simulink model "MX_Gateway"  (Simulink 9.0) (continued)
  CD_EOT_DEBOUNCE_LOW = Bobcat.Parameter; CreateAliasCD( CD_EOT_DEBOUNCE_LOW                                  ,uint16(2)                  ,'ConstantDataPointer' ,'CONSTANT_DATA' ,'EOT_DEBOUNCE_LOW')    [cdata.m]    (also in hc12_cdata.m; m4_cdata.m)
  CD_EOT_DEBOUNCE_NORMAL = Bobcat.Parameter; CreateAliasCD( CD_EOT_DEBOUNCE_NORMAL                               ,uint16(2)                  ,'ConstantDataPointer' ,'CONSTANT_DATA' ,'EOT_DEBOUNCE_NORMAL')    [cdata.m]    (also in hc12_cdata.m; m4_cdata.m)
  CD_EOT_EXTREMELY_LOW = Bobcat.Parameter; CreateAliasCD( CD_EOT_EXTREMELY_LOW                                 ,uint16(81)                 ,'ConstantDataPointer' ,'CONSTANT_DATA' ,'EOT_EXTREMELY_LOW')    [cdata.m]    (also in hc12_cdata.m; m4_cdata.m)
  CD_EOT_FUNCTION = Bobcat.Parameter; CreateAliasCD( CD_EOT_FUNCTION                                      ,uint8(255)                 ,'ConstantDataPointer' ,'CONSTANT_DATA' ,'EOT_FUNCTION')    [cdata.m]    (also in hc12_cdata.m; m4_cdata.m)
  CD_EOT_LOW = Bobcat.Parameter; CreateAliasCD( CD_EOT_LOW                                           ,uint16(90)                 ,'ConstantDataPointer' ,'CONSTANT_DATA' ,'EOT_LOW')    [cdata.m]    (also in hc12_cdata.m; m4_cdata.m)
  CD_EOT_TEMPERATURE_HYSTERESIS = Bobcat.Parameter; CreateAliasCD( CD_EOT_TEMPERATURE_HYSTERESIS                        ,uint16(2)                  ,'ConstantDataPointer' ,'CONSTANT_DATA' ,'EOT_TEMPERATURE_HYSTERESIS')    [cdata.m]    (also in hc12_cdata.m; m4_cdata.m)
  CD_FAST_TRAVEL_FUNCTION = Bobcat.Parameter; CreateAliasCD( CD_FAST_TRAVEL_FUNCTION                              ,uint8(255)                 ,'ConstantDataPointer' ,'CONSTANT_DATA' ,'FAST_TRAVEL_FUNCTION')    [cdata.m]    (also in hc12_cdata.m; m4_cdata.m)
  CD_FAST_TRAVEL_THROTTLE_DELAY = Bobcat.Parameter; CreateAliasCD( CD_FAST_TRAVEL_THROTTLE_DELAY                        ,uint16(10)                 ,'ConstantDataPointer' ,'CONSTANT_DATA' ,'FAST_TRAVEL_THROTTLE_DELAY')    [cdata.m]    (also in hc12_cdata.m; m4_cdata.m)
  CD_FAST_TRAVEL_THROTTLE_LIMIT = Bobcat.Parameter; CreateAliasCD( CD_FAST_TRAVEL_THROTTLE_LIMIT                        ,uint16(1000)               ,'ConstantDataPointer' ,'CONSTANT_DATA' ,'FAST_TRAVEL_THROTTLE_LIMIT')    [cdata.m]    (also in hc12_cdata.m; m4_cdata.m)
  CD_FLYWHEEL_TEETH = Bobcat.Parameter; CreateAliasCD( CD_FLYWHEEL_TEETH                                    ,uint8(83)                  ,'ConstantDataPointer' ,'CONSTANT_DATA' ,'FLYWHEEL_TEETH')    [cdata.m]    (also in hc12_cdata.m; m4_cdata.m)
  CD_FUEL_LEVEL_FILTER_FAST_VALUE = Bobcat.Parameter; CreateAliasCD( CD_FUEL_LEVEL_FILTER_FAST_VALUE                      ,uint8(5)                   ,'ConstantDataPointer' ,'CONSTANT_DATA' ,'FUEL_LEVEL_FILTER_FAST_VALUE')    [cdata.m]    (also in hc12_cdata.m; m4_cdata.m)
  CD_FUEL_LEVEL_FILTER_SLOW_VALUE = Bobcat.Parameter; CreateAliasCD( CD_FUEL_LEVEL_FILTER_SLOW_VALUE                      ,uint8(10)                  ,'ConstantDataPointer' ,'CONSTANT_DATA' ,'FUEL_LEVEL_FILTER_SLOW_VALUE')    [cdata.m]    (also in hc12_cdata.m; m4_cdata.m)
  CD_FUEL_PRIMARY_FEEDBACK_FUNCTION = Bobcat.Parameter; CreateAliasCD( CD_FUEL_PRIMARY_FEEDBACK_FUNCTION                    ,uint8(0)                   ,'ConstantDataPointer' ,'CONSTANT_DATA' ,'FUEL_PRIMARY_FEEDBACK_FUNCTION')    [cdata.m]    (also in hc12_cdata.m; m4_cdata.m)
  ... (+246 more)
undefined symbols (not defined in the file or in its folder): sigbuilder_block
undefined symbols (not defined in the file): CD_FUEL_RESOLUTION_TIMER
libraries: simulink x186, MX_Library x107
(part 42 of 48)

== stateflow chart MX_Gtwy_Control/PanelInterface/InformationDisplay/Display_Type ==
  state BUTTON_STATE [parallel]
    settings: executionOrder=1
    state INFO_BUTTON [parallel]
      settings: executionOrder=1
      state INACTIVE
        en:state_InfoButton = INACTIVE;
      state EDGE
        en:debounceButton = 0;
        du:debounceButton++;
      state INACTIVE_WAIT
        en:state_InfoButton = INACTIVE;
      state ACTIVE
        en:state_InfoButton = ACTIVE;
      (default) -> BUTTON_STATE.INFO_BUTTON.INACTIVE
      BUTTON_STATE.INFO_BUTTON.INACTIVE -> BUTTON_STATE.INFO_BUTTON.EDGE : [((msg_PanelButtonState[0] & INFO_BUTTON_MASK)  == INFO_BUTTON_PRESS)]
      BUTTON_STATE.INFO_BUTTON.ACTIVE -> BUTTON_STATE.INFO_BUTTON.INACTIVE : [((msg_PanelButtonState[0] & INFO_BUTTON_MASK)  == INACTIVE)]
      BUTTON_STATE.INFO_BUTTON.INACTIVE_WAIT -> BUTTON_STATE.INFO_BUTTON.INACTIVE : [((msg_PanelButtonState[0] & INFO_BUTTON_MASK)  == INACTIVE)]
      BUTTON_STATE.INFO_BUTTON.EDGE -> BUTTON_STATE.INFO_BUTTON.INACTIVE_WAIT [order 1] : [(debounceButton > 100)...
          |      || (state_Jump == ACTIVE)]
      BUTTON_STATE.INFO_BUTTON.EDGE -> BUTTON_STATE.INFO_BUTTON.ACTIVE [order 2] : [((msg_PanelButtonState[0] & INFO_BUTTON_MASK)  == INACTIVE)...
          | && (state_Jump != ACTIVE)]
    state MOVE_RIGHT [parallel]
      settings: executionOrder=2
      state PANEL_BUTTON [parallel]
        settings: executionOrder=1
        state INACTIVE
          en:state_RightButton = INACTIVE;
        state EDGE
          en:debounceRight = 0;
          du:debounceRight++;
        state INACTIVE_WAIT
          en:state_RightButton = INACTIVE;
        state ACTIVE
          en:state_RightButton = ACTIVE;
        (default) -> BUTTON_STATE.MOVE_RIGHT.PANEL_BUTTON.INACTIVE
        BUTTON_STATE.MOVE_RIGHT.PANEL_BUTTON.INACTIVE -> BUTTON_STATE.MOVE_RIGHT.PANEL_BUTTON.EDGE : [((msg_PanelButtonState[0] & RIGHT_BUTTON_MASK) == RIGHT_BUTTON_PRESS)...
            | && (enable_RightLeft)]
        BUTTON_STATE.MOVE_RIGHT.PANEL_BUTTON.ACTIVE -> BUTTON_STATE.MOVE_RIGHT.PANEL_BUTTON.INACTIVE : [((msg_PanelButtonState[0] & RIGHT_BUTTON_MASK)  == INACTIVE)]
        BUTTON_STATE.MOVE_RIGHT.PANEL_BUTTON.INACTIVE_WAIT -> BUTTON_STATE.MOVE_RIGHT.PANEL_BUTTON.INACTIVE : [((msg_PanelButtonState[0] & RIGHT_BUTTON_MASK)  == INACTIVE)]
        BUTTON_STATE.MOVE_RIGHT.PANEL_BUTTON.EDGE -> BUTTON_STATE.MOVE_RIGHT.PANEL_BUTTON.INACTIVE_WAIT [order 1] : [(debounceRight > 100)]
        BUTTON_STATE.MOVE_RIGHT.PANEL_BUTTON.EDGE -> BUTTON_STATE.MOVE_RIGHT.PANEL_BUTTON.ACTIVE [order 2] : [((msg_PanelButtonState[0] & RIGHT_BUTTON_MASK)  == INACTIVE)]
    state MOVE_LEFT [parallel]
      settings: executionOrder=3
      state PANEL_BUTTON [parallel]
        settings: executionOrder=1
        state INACTIVE
          en:state_LeftButton = INACTIVE;
        state EDGE
          en:debounceLeft = 0;
          du:debounceLeft++;
        state INACTIVE_WAIT
          en:state_LeftButton = INACTIVE;
        state ACTIVE
          en:state_LeftButton = ACTIVE;
        (default) -> BUTTON_STATE.MOVE_LEFT.PANEL_BUTTON.INACTIVE
        BUTTON_STATE.MOVE_LEFT.PANEL_BUTTON.INACTIVE -> BUTTON_STATE.MOVE_LEFT.PANEL_BUTTON.EDGE : [((msg_PanelButtonState[0] & LEFT_BUTTON_MASK) == LEFT_BUTTON_PRESS)...
            | && (enable_RightLeft)]
        BUTTON_STATE.MOVE_LEFT.PANEL_BUTTON.ACTIVE -> BUTTON_STATE.MOVE_LEFT.PANEL_BUTTON.INACTIVE : [((msg_PanelButtonState[0] & LEFT_BUTTON_MASK)  == INACTIVE)]
        BUTTON_STATE.MOVE_LEFT.PANEL_BUTTON.INACTIVE_WAIT -> BUTTON_STATE.MOVE_LEFT.PANEL_BUTTON.INACTIVE : [((msg_PanelButtonState[0] & LEFT_BUTTON_MASK)  == INACTIVE)]
        BUTTON_STATE.MOVE_LEFT.PANEL_BUTTON.EDGE -> BUTTON_STATE.MOVE_LEFT.PANEL_BUTTON.INACTIVE_WAIT [order 1] : [(debounceLeft > 100)]
        BUTTON_STATE.MOVE_LEFT.PANEL_BUTTON.EDGE -> BUTTON_STATE.MOVE_LEFT.PANEL_BUTTON.ACTIVE [order 2] : [((msg_PanelButtonState[0] & LEFT_BUTTON_MASK)  == INACTIVE)]
  state PRIMARY_STATE [parallel]
    settings: executionOrder=3
    state ACTIVE
      on after (50,tick): prev_SecondAux = INACTIVE;
      (history)
      state ENGINE_HOURS
        en:state_InfoPrimary = DISPLAY_ENGINE_HOURS;
        en:state_InfoButton = INACTIVE;
        en:enable_RightLeft = INACTIVE;
        state RESET_STATE
          en:
          en:state_Jump = ACTIVE;
        state INACTIVE
          en:state_Jump = 0;
        state DEBOUNCE
          en:debounceCount = 0;
          du:debounceCount++;
        (default) -> PRIMARY_STATE.ACTIVE.ENGINE_HOURS.INACTIVE
        PRIMARY_STATE.ACTIVE.ENGINE_HOURS.INACTIVE -> PRIMARY_STATE.ACTIVE.ENGINE_HOURS.DEBOUNCE : [((msg_PanelButtonState[0] & INFO_BUTTON_MASK)  == INFO_BUTTON_PRESS)...
            | && (state_InfoSecondary == INACTIVE)]
        PRIMARY_STATE.ACTIVE.ENGINE_HOURS.DEBOUNCE -> PRIMARY_STATE.ACTIVE.ENGINE_HOURS.INACTIVE [order 2] : [((msg_PanelButtonState[0] & INFO_BUTTON_MASK)  == INACTIVE)]
        PRIMARY_STATE.ACTIVE.ENGINE_HOURS.DEBOUNCE -> PRIMARY_STATE.ACTIVE.ENGINE_HOURS.RESET_STATE [order 1] : [(debounceCount >= 50)]
      state JOB_CLOCK_1
        en:state_InfoPrimary = DISPLAY_JOB_CLOCK_1;
        en:state_InfoButton = INACTIVE;
        en:enable_RightLeft = INACTIVE;
        state RESET_STATE
          en:
          en:state_Jump = ACTIVE;
        state INACTIVE
          en:state_Jump = 0;
        state DEBOUNCE
          en:debounceCount = 0;
          du:debounceCount++;
        (default) -> PRIMARY_STATE.ACTIVE.JOB_CLOCK_1.INACTIVE
        PRIMARY_STATE.ACTIVE.JOB_CLOCK_1.DEBOUNCE -> PRIMARY_STATE.ACTIVE.JOB_CLOCK_1.INACTIVE [order 2] : [((msg_PanelButtonState[0] & INFO_BUTTON_MASK)  == INACTIVE)]
        PRIMARY_STATE.ACTIVE.JOB_CLOCK_1.DEBOUNCE -> PRIMARY_STATE.ACTIVE.JOB_CLOCK_1.RESET_STATE [order 1] : [(debounceCount >= 25)]
        PRIMARY_STATE.ACTIVE.JOB_CLOCK_1.INACTIVE -> PRIMARY_STATE.ACTIVE.JOB_CLOCK_1.DEBOUNCE : [((msg_PanelButtonState[0] & INFO_BUTTON_MASK)  == INFO_BUTTON_PRESS)...
            | && (state_InfoSecondary == INACTIVE)]
      state JOB_CLOCK_RESET1
        en:
        ee_JobClockLHP[0] = 0;
        matlab_UpdateEEVar();
      state JOB_CLOCK_2
        en:state_InfoPrimary = DISPLAY_JOB_CLOCK_2;
        en:state_InfoButton = INACTIVE;
        en:enable_RightLeft = INACTIVE;
        state RESET_STATE
          en:
          en:state_Jump = ACTIVE;
        state INACTIVE
          en:state_Jump = 0;
        state DEBOUNCE
          en:debounceCount = 0;
          du:debounceCount++;
        (default) -> PRIMARY_STATE.ACTIVE.JOB_CLOCK_2.INACTIVE
        PRIMARY_STATE.ACTIVE.JOB_CLOCK_2.DEBOUNCE -> PRIMARY_STATE.ACTIVE.JOB_CLOCK_2.INACTIVE [order 2] : [((msg_PanelButtonState[0] & INFO_BUTTON_MASK)  == INACTIVE)]
        PRIMARY_STATE.ACTIVE.JOB_CLOCK_2.DEBOUNCE -> PRIMARY_STATE.ACTIVE.JOB_CLOCK_2.RESET_STATE [order 1] : [(debounceCount >= 25)]
        PRIMARY_STATE.ACTIVE.JOB_CLOCK_2.INACTIVE -> PRIMARY_STATE.ACTIVE.JOB_CLOCK_2.DEBOUNCE : [((msg_PanelButtonState[0] & INFO_BUTTON_MASK)  == INFO_BUTTON_PRESS)...
            | && (state_InfoSecondary == INACTIVE)]
      state JOB_CLOCK_RESET2
        en:
        ee_JobClockLHP[1] = 0;
        matlab_UpdateEEVar();
      state FACTORY
        en:
        state_InfoButton = INACTIVE;
        state_Jump = INACTIVE;
        state HOT
          en:
          state_InfoPrimary = DISPLAY_HOT;
        state ECT
          en:
          state_InfoPrimary = DISPLAY_ECT;
        (default) -> (junction 4982)
        (junction 4982) -> HOT [order 1] : [CD_HOT_FUNCTION != NON_FUNCTIONAL]
        (junction 4982) -> PRIMARY_STATE.ACTIVE.FACTORY.ECT [order 2]
      state ENGINE_RPM
        en:state_InfoPrimary = DISPLAY_ENGINE_RPM;
        en:state_InfoButton = INACTIVE;
      state EMEA_CONTROLS1
        en:state_InfoPrimary = DISPLAY_EMEA_CONTROLS;
      state BATTERY_VOLTAGE
        en:state_InfoPrimary = DISPLAY_BATTERY_VOLTAGE;
        en:state_InfoButton = INACTIVE;
      state SERVICE_CLOCK_RESET
        en:state_InfoPrimary = DISPLAY_SERVICE_CLOCK_RESET;
      state SERVICE_CLOCK
        en:state_InfoPrimary = DISPLAY_SERVICE_CLOCK;
        en:state_InfoButton = INACTIVE;
        en:enable_RightLeft = INACTIVE;
        state INACTIVE
          en:state_Jump = 0;
        state JUMP_STATE
          en:state_Jump = ACTIVE;
        state DEBOUNCE
          en:debounceCount = 0;
          du:debounceCount++;
        (default) -> PRIMARY_STATE.ACTIVE.SERVICE_CLOCK.INACTIVE
        PRIMARY_STATE.ACTIVE.SERVICE_CLOCK.INACTIVE -> PRIMARY_STATE.ACTIVE.SERVICE_CLOCK.DEBOUNCE : [((msg_PanelButtonState[0] & INFO_BUTTON_MASK)  == INFO_BUTTON_PRESS)...
            | && (state_ServiceClock == ACTIVE)...
            | && (state_InfoSecondary == INACTIVE)]
        PRIMARY_STATE.ACTIVE.SERVICE_CLOCK.DEBOUNCE -> PRIMARY_STATE.ACTIVE.SERVICE_CLOCK.INACTIVE [order 2] : [((msg_PanelButtonState[0] & INFO_BUTTON_MASK)  == INACTIVE)]
        PRIMARY_STATE.ACTIVE.SERVICE_CLOCK.DEBOUNCE -> JUMP_STATE [order 1] : [(debounceCount >= 70)]
      state ERROR_CODES
        en:state_InfoPrimary = DISPLAY_ERROR_CODES;
        en:state_InfoButton = INACTIVE;
      state EOP
        en:state_InfoPrimary = DISPLAY_EOP;
        en:state_InfoButton = INACTIVE;
      state ECT
        en:state_InfoPrimary = DISPLAY_ECT;
        en:state_InfoButton = INACTIVE;
      state HOT2
        en:state_InfoPrimary = DISPLAY_HOT;
        en:state_InfoButton = INACTIVE;
      (default) -> ENGINE_HOURS
      JOB_CLOCK_1 -> JOB_CLOCK_RESET1 [order 1] : [(state_Jump == ACTIVE)]
      ENGINE_HOURS -> JOB_CLOCK_1 [order 2] : [(state_InfoButton == ACTIVE)]
      JOB_CLOCK_RESET1 -> JOB_CLOCK_1 : after(10,tick)
      JOB_CLOCK_1 -> JOB_CLOCK_2 [order 2] : [(state_InfoButton == ACTIVE)]
      (junction 4943) -> ENGINE_HOURS
      ENGINE_HOURS -> ENGINE_RPM [order 3] : [state_Engine == ENGINE_CRANKING]
      ENGINE_HOURS -> FACTORY [order 1] : [(state_Jump == ACTIVE)]
      FACTORY -> ENGINE_HOURS : [(state_InfoButton == ACTIVE)]
      JOB_CLOCK_2 -> JOB_CLOCK_RESET2 [order 2] : [(state_Jump == ACTIVE)]
      JOB_CLOCK_RESET2 -> JOB_CLOCK_2 : after(10,tick)
      JOB_CLOCK_2 -> ENGINE_RPM [order 1] : [(state_InfoButton == ACTIVE)]
      ENGINE_RPM -> EMEA_CONTROLS1 [order 1] : [(state_InfoButton == ACTIVE)...
          | && (CD_EMEA_CONTROLS_DISPLAY_MODE != NON_FUNCTIONAL)]
      ENGINE_RPM -> BATTERY_VOLTAGE [order 2] : [(state_InfoButton == ACTIVE)]
      EMEA_CONTROLS1 -> BATTERY_VOLTAGE : [(state_InfoButton == ACTIVE)]
      BATTERY_VOLTAGE -> SERVICE_CLOCK : [(state_InfoButton == ACTIVE)]
      SERVICE_CLOCK -> SERVICE_CLOCK_RESET [order 1] : [(state_Jump == ACTIVE)]
      SERVICE_CLOCK_RESET -> SERVICE_CLOCK : after(70,tick)
      SERVICE_CLOCK -> PRIMARY_STATE.ACTIVE.ERROR_CODES [order 2] : [(state_InfoButton == ACTIVE)]
      PRIMARY_STATE.ACTIVE.ERROR_CODES -> (junction 4943) [order 2] : [(state_InfoButton == ACTIVE)]
      (junction 4944) -> (junction 4943)
      PRIMARY_STATE.ACTIVE.ERROR_CODES -> EOP [order 1] : [(ee_EngData == 1)...
          | && (state_InfoButton == ACTIVE)]
      HOT2 -> (junction 4944) : [(state_InfoButton == ACTIVE)]
      EOP -> PRIMARY_STATE.ACTIVE.ECT : [(state_InfoButton == ACTIVE)]
      PRIMARY_STATE.ACTIVE.ECT -> HOT2 : [(state_InfoButton == ACTIVE)]
    state SECOND_AUX
      en:state_InfoPrimary = DISPLAY_SECOND_AUX;
      prev_InfoSecondary = state_InfoSecondary;
      prev_SecondAux = ACTIVE;
    note: Info Button press should keep us in primary state
        | for 5 seconds, if second aux just turns off we
        | should be able to get out of primary display
        | right away if it turns back on
    state INACTIVE
      en:
      state_InfoPrimary = DISPLAY_PRIMARY_INACTIVE;
      prev_InfoSecondary = state_InfoSecondary;
    state ERROR_CODES
      en:
      state_InfoPrimary = DISPLAY_ERROR_CODES;
      state_InfoButton = INACTIVE;
      debounceCode = 0;
      du:
      debounceCode++;
      ex:
      flag_StdActiveWarn = INACTIVE;
    state EMEA_CONTROLS
      en:state_InfoPrimary = DISPLAY_EMEA_CONTROLS;
      prev_InfoSecondary = state_InfoSecondary;
    (default) -> PRIMARY_STATE.ACTIVE : {
        | prev_InfoSecondary = INACTIVE;
        | prev_SecondAux = INACTIVE;
        | }
    PRIMARY_STATE.ACTIVE -> SECOND_AUX [order 4] : [CD_SECOND_AUX_FUNCTION != NON_FUNCTIONAL...
        | && (cmd_SecondAux == ACTIVE)...
        | && (prev_SecondAux == INACTIVE)]
    SECOND_AUX -> PRIMARY_STATE.ACTIVE [order 2] : [state_InfoButton == ACTIVE]
    SECOND_AUX -> PRIMARY_STATE.INACTIVE [order 1] : [(state_InfoSecondary != INACTIVE)...
        | && (state_InfoSecondary != prev_InfoSecondary)]
    SECOND_AUX -> PRIMARY_STATE.ACTIVE [order 3] : [cmd_SecondAux == INACTIVE]
        | {prev_SecondAux = INACTIVE;}
    PRIMARY_STATE.INACTIVE -> SECOND_AUX [order 2] : [(cmd_SecondAux == ACTIVE)...
        |  && (prev_SecondAux == ACTIVE)]
    PRIMARY_STATE.ACTIVE -> PRIMARY_STATE.INACTIVE [order 1] : [(state_InfoSecondary != INACTIVE)...
        | && ((state_InfoSecondary != prev_InfoSecondary) || after(40, tick))]
    EMEA_CONTROLS -> PRIMARY_STATE.INACTIVE [order 5] : [(state_InfoSecondary != INACTIVE)...
        | && (state_InfoSecondary != prev_InfoSecondary)]
    PRIMARY_STATE.ERROR_CODES -> PRIMARY_STATE.INACTIVE [order 2] : [(state_InfoSecondary != INACTIVE)...
        | && ((state_InfoSecondary != prev_InfoSecondary) || after(40, tick))]
    PRIMARY_STATE.INACTIVE -> PRIMARY_STATE.ACTIVE [order 1] : [(state_InfoSecondary == INACTIVE)...
        | || ((state_InfoButton == ACTIVE) && (state_InfoSecondary != DISPLAY_DELUXE_ERROR) && (state_InfoSecondary != DISPLAY_SHUTDOWN)...
        | && (state_InfoSecondary != DISPLAY_SERVICE_CLOCK_RESET) && (state_InfoSecondary != DISPLAY_SERVICE_CLOCK_ALARM))]
    PRIMARY_STATE.ACTIVE -> PRIMARY_STATE.ERROR_CODES [order 2] : [(CD_AUTO_DISPLAY_FAULT_FUNCTION != NON_FUNCTIONAL)...
        | && (flag_StdActiveWarn)...
        | && (ee_DeluxeG5 != ACTIVE)...
        | && CD_DELUXE_GEN5_5_FUNCTION == NON_FUNCTIONAL]
    PRIMARY_STATE.ERROR_CODES -> PRIMARY_STATE.ACTIVE [order 1] : [(debounceCode >= CD_AUTO_DISPLAY_FAULT_DEBOUNCE_ACTIVE)...
        | || (state_InfoButton == ACTIVE)]
    EMEA_CONTROLS -> PRIMARY_STATE.ERROR_CODES [order 6] : [(CD_AUTO_DISPLAY_FAULT_FUNCTION != NON_FUNCTIONAL)...
        | && (flag_StdActiveWarn)...
        | && (ee_DeluxeG5 != ACTIVE)...
        | && CD_DELUXE_GEN5_5_FUNCTION == NON_FUNCTIONAL]
    PRIMARY_STATE.ACTIVE -> EMEA_CONTROLS [order 3] : [(CD_EMEA_CONTROLS_DISPLAY_MODE != NON_FUNCTIONAL)...
        | && mode_ControlChange...
        | && state_InfoPrimary != DISPLAY_EMEA_CONTROLS]
    EMEA_CONTROLS -> PRIMARY_STATE.ACTIVE [order 3] : [state_InfoButton == ACTIVE]
    EMEA_CONTROLS -> PRIMARY_STATE.ACTIVE [order 4] : [after(CD_EMEA_CONTROLS_DISPLAY_TIME, tick)]
    EMEA_CONTROLS -> PRIMARY_STATE.ACTIVE [order 1] : [mode_LeftControl == MODE_LEFT_NONE...
        | && mode_RightControl == MODE_RIGHT_NONE]
    EMEA_CONTROLS -> EMEA_CONTROLS [order 2] : [mode_ControlChange]
  state SECONDARY_STATE [parallel]
    settings: executionOrder=2
    state DELUXE_GOOD
      state VERSION
        en:state_InfoSecondary = DISPLAY_VERSION;
      state NON_SHUTDOWN
        state FLASH_COLD
          en:state_InfoSecondary = DISPLAY_FLASH_COLD;
        state COLD
          en:state_InfoSecondary = DISPLAY_COLD;
        state SERVICE_CLOCK_ALARM
          en:state_InfoSecondary = DISPLAY_SERVICE_CLOCK_ALARM;
          en:serviceClockAlarmTimer = 0;
          du:serviceClockAlarmTimer++;
        state NO_STATE
          en:state_InfoSecondary = INACTIVE;
        state GLOW_PLUGS
          en:state_InfoSecondary = DISPLAY_GLOW_PLUGS;
        state THROTTLE_CAL_DIAGNOSTICS
          en:
          state_InfoSecondary = DISPLAY_CAN_THROTTLE_DIAG;
        state OFFSET
          en:state_InfoSecondary = DISPLAY_OFFSET;
        state Aux_Detent_Error
          state HOLD
            en:
            state_InfoSecondary = DISPLAY_HOLD;
          state AUX
            en:
            state_InfoSecondary = DISPLAY_AUX;
          (default) -> HOLD
          AUX -> HOLD : [after(CD_AUX_DETENT_CYCLE_TIME,tick)]
          HOLD -> AUX : [after(CD_AUX_DETENT_CYCLE_TIME,tick)]
        COLD -> FLASH_COLD [order 2] : [(state_TC == EVENT)]
        FLASH_COLD -> COLD : [(state_TC != EVENT)]
        COLD -> NO_STATE [order 1] : [(state_TC == NORMAL)]
        NO_STATE -> COLD [order 1] : [(state_TC == LOW)...
            | || (state_TC == EXTREMELY_LOW)]
        (default) -> NO_STATE
        NO_STATE -> GLOW_PLUGS [order 2] : [(count_GlowPlug > 0)]
        NO_STATE -> SERVICE_CLOCK_ALARM [order 3] : [(state_ServiceClock ==ACTIVE)...
            | && (serviceClockAlarmTimer == 0)...
            | && (state_MachineLock != ACTIVE)]
        GLOW_PLUGS -> NO_STATE : [(count_GlowPlug == 0)...
            | || ((state_CANThrottleCalDiag != INACTIVE)...
            | && (state_InfoPrimary == DISPLAY_ENGINE_HOURS))]
        SERVICE_CLOCK_ALARM -> NO_STATE : [(serviceClockAlarmTimer >=...
            |  CD_DISPLAY_SERVICE_CLOCK_ALARM_TIME)]
        NO_STATE -> THROTTLE_CAL_DIAGNOSTICS [order 5] : [(state_CANThrottleCalDiag != INACTIVE)...
            | && (state_InfoPrimary == DISPLAY_ENGINE_HOURS)]
        THROTTLE_CAL_DIAGNOSTICS -> NO_STATE : [after(CD_CAN_THROTTLE_CAL_DIAG_TIME, tick)...
            | || (state_CANThrottleCalDiag == INACTIVE)]
        NO_STATE -> OFFSET [order 6] : [CD_SECOND_AUX_FUNCTION != NON_FUNCTIONAL...
            | && fallingedge_SecondAux]
        OFFSET -> NO_STATE : [after(CD_SECOND_AUX_OFFSET_DISP_TIME, tick)]
        NO_STATE -> Aux_Detent_Error [order 4] : [state_Detent == ERR_DETENT...
            | && ee_DeluxeG5 != ACTIVE...
            | && CD_DELUXE_GEN5_5_FUNCTION == NON_FUNCTIONAL]
        Aux_Detent_Error -> NO_STATE : [after(CD_AUX_DETENT_FLASH_TIME, tick)...
            | || state_DetentEnable == ACTIVE]
      state KSS
        en: state_InfoSecondary = KSSDisplayState;
        du: state_InfoSecondary = KSSDisplayState;
      state SHUTDOWN
        en:state_InfoSecondary = DISPLAY_SHUTDOWN;
        en:shutdownTimer = 0;
        du:shutdownTimer++;
      (default) -> NON_SHUTDOWN
      NON_SHUTDOWN -> VERSION [order 2] : [(state_LightButton == ACTIVE)]
      NON_SHUTDOWN -> KSS [order 3] : [(KSSDisplayState != INACTIVE)]
      VERSION -> NON_SHUTDOWN : [(state_Engine == SHUTDOWN)...
          | || (state_LightButton != ACTIVE)]
      KSS -> NON_SHUTDOWN : [(KSSDisplayState == INACTIVE)]
      SHUTDOWN -> NON_SHUTDOWN : [(shutdownTimer >= CD_DISPLAY_SHUTDOWN_TIME)]
      NON_SHUTDOWN -> SHUTDOWN [order 1] : [(state_Engine == SHUTDOWN)...
          | && (shutdownTimer == 0)...
          | && (state_Key != MULTIPLE)]
    state DELUXE_ERROR
      en:state_InfoSecondary = DISPLAY_DELUXE_ERROR;
    (default) -> DELUXE_GOOD
    DELUXE_ERROR -> DELUXE_GOOD : [(state_KeylessPanel != NO_COMMUNICATION)]
    DELUXE_GOOD -> DELUXE_ERROR : [(state_KeylessPanel == NO_COMMUNICATION)]
  state LIGHT_BUTTON_STATE [parallel]
    settings: executionOrder=4
    state INACTIVE
      en:state_LightButton = INACTIVE;
    state EDGE
      en:debounceCount = 0;
      du:debounceCount++;
    state ACTIVE
      en:state_LightButton = ACTIVE;
      en:debounceCount = 0;
      du:debounceCount++;
    (default) -> LIGHT_BUTTON_STATE.INACTIVE
    LIGHT_BUTTON_STATE.INACTIVE -> LIGHT_BUTTON_STATE.EDGE : [((msg_PanelButtonState[0] & LIGHT_BUTTON_MASK)  == LIGHT_BUTTON_PRESS)]
    LIGHT_BUTTON_STATE.EDGE -> LIGHT_BUTTON_STATE.INACTIVE [order 2] : [((msg_PanelButtonState[0] & LIGHT_BUTTON_MASK) == INACTIVE)]
    LIGHT_BUTTON_STATE.ACTIVE -> LIGHT_BUTTON_STATE.INACTIVE : [(debounceCount >= 100)]
    LIGHT_BUTTON_STATE.EDGE -> LIGHT_BUTTON_STATE.ACTIVE [order 1] : [(debounceCount >= 60)]
  data: state_InfoPrimary output uint8; debounceCount local uint16; count_GlowPlug input uint16; ACTIVE parameter uint8; DISPLAY_BATTERY_VOLTAGE parameter uint8; DISPLAY_ENGINE_HOURS parameter uint8; DISPLAY_ENGINE_RPM parameter uint8; DISPLAY_ERROR_CODES parameter uint8; DISPLAY_GLOW_PLUGS parameter uint8; DISPLAY_SECOND_AUX parameter uint8; INACTIVE parameter uint8; msg_PanelButtonState input uint8 size=8; state_ServiceClock input uint8; serviceClockAlarmTimer local uint16 =0; CD_DISPLAY_SERVICE_CLOCK_ALARM_TIME parameter uint16; DISPLAY_SERVICE_CLOCK_ALARM parameter uint8; state_Engine input uint8; shutdownTimer local uint16 =0; CD_DISPLAY_SHUTDOWN_TIME parameter uint16; SHUTDOWN parameter uint8; DISPLAY_SHUTDOWN parameter uint8; DISPLAY_SERVICE_CLOCK_RESET parameter uint8; state_Key input uint8; MULTIPLE parameter uint8; state_InfoButton output uint8; state_InfoSecondary output uint8; state_Jump local uint8 =0; state_LightButton local uint8; DISPLAY_VERSION parameter uint8; DISPLAY_DELUXE_ERROR parameter uint8; NO_COMMUNICATION parameter uint8; INFO_BUTTON_MASK parameter uint8; INFO_BUTTON_PRESS parameter uint8; LIGHT_BUTTON_MASK parameter uint8; LIGHT_BUTTON_PRESS parameter uint8; debounceButton local uint16; DISPLAY_EOP parameter uint8; DISPLAY_ECT parameter uint8; DISPLAY_HOT parameter uint8; ee_EngData data_store_memory double; debounceLeft local uint16; LEFT_BUTTON_MASK parameter uint8; RIGHT_BUTTON_PRESS parameter uint8; debounceRight local uint16; state_LeftButton output uint8; state_RightButton output uint8; RIGHT_BUTTON_MASK parameter uint8; LEFT_BUTTON_PRESS parameter uint8; enable_RightLeft output uint8; NON_FUNCTIONAL parameter uint8; state_Detent input uint8; CD_AUX_DETENT_FLASH_TIME parameter uint16; ERR_DETENT parameter uint8; DISPLAY_HOLD parameter uint8; DISPLAY_AUX parameter uint8; CD_AUX_DETENT_CYCLE_TIME parameter uint16; state_DetentEnable input uint8; ee_JobClockLHP data_store_memory double; DISPLAY_JOB_CLOCK_1 parameter uint8; DISPLAY_JOB_CLOCK_2 parameter uint8; NORMAL parameter uint8; DISPLAY_CAN_THROTTLE_DIAG parameter uint8; CD_CAN_THROTTLE_CAL_DIAG_TIME parameter uint16; prev_InfoSecondary local uint8; DISPLAY_PRIMARY_INACTIVE parameter uint8; ENGINE_CRANKING parameter uint8; state_CANThrottleCalDiag input uint8; ee_DeluxeG5 data_store_memory double; CD_HOT_FUNCTION parameter uint16; state_TC input uint8; LOW parameter uint8; EXTREMELY_LOW parameter uint8; EVENT parameter uint8; DISPLAY_COLD parameter uint8; DISPLAY_FLASH_COLD parameter uint8; CD_AUTO_DISPLAY_FAULT_FUNCTION parameter uint8; CD_AUTO_DISPLAY_FAULT_DEBOUNCE_ACTIVE parameter uint16; flag_StdActiveWarn data_store_memory double; debounceCode local uint16; KSSDisplayState input uint8; state_KeylessPanel input uint8; state_MachineLock input uint8; cmd_SecondAux input boolean; prev_SecondAux local boolean; fallingedge_SecondAux input boolean; CD_SECOND_AUX_OFFSET_DISP_TIME parameter uint16; DISPLAY_OFFSET parameter uint8; DISPLAY_SERVICE_CLOCK parameter uint8; CD_DELUXE_GEN5_5_FUNCTION parameter uint8; CD_AUX_FUNCTION parameter uint8; AUX_EMEA_CONTROLS parameter uint8; mode_ControlChange input boolean; CD_EMEA_CONTROLS_DISPLAY_TIME parameter uint16; CD_SECOND_AUX_FUNCTION parameter uint8; DISPLAY_EMEA_CONTROLS parameter uint8; mode_LeftControl input uint8; mode_RightControl input uint8; MODE_LEFT_NONE parameter uint8; MODE_RIGHT_NONE parameter uint8; CD_EMEA_CONTROLS_DISPLAY_MODE parameter uint8
